simulink model "CDF_VSVPWM_Grid_Parallel"  (R2024b) (continued)
== matlab function CDF_VSVPWM_Grid_Parallel/VSVPWM/矢量作用时间计算/I3/sin(pi//6+u) ==
  data: u input; y output
function y = fcn(u)

y = sin(pi/6+u);

== matlab function CDF_VSVPWM_Grid_Parallel/VSVPWM/矢量作用时间计算/I4/sin(pi//3-u) ==
  data: u input; y output
function y = fcn(u)

y = sin(pi/3-u);

== matlab function CDF_VSVPWM_Grid_Parallel/VSVPWM/矢量作用时间计算/I4/sin(pi//6+u) ==
  data: u input; y output
function y = fcn(u)

y = sin(pi/6+u);

== matlab function CDF_VSVPWM_Grid_Parallel/VSVPWM/矢量作用时间计算/I5/sin(pi//3+u) ==
  data: u input; y output
function y = fcn(u)

y = sin(pi/3+u);

== matlab function CDF_VSVPWM_Grid_Parallel/VSVPWM/矢量作用时间计算/I5/sin(pi//6+u) ==
  data: u input; y output
function y = fcn(u)

y = sin(pi/6+u);

== matlab function CDF_VSVPWM_Grid_Parallel/VSVPWM/MATLAB Function ==
  data: n input; T1 output; N input; Ta input; Tb input; Tc input; T2 output; T3 output; T4 output; T5 output; k1 input; k2 input
function [T1,T2,T3,T4,T5] = fcn(n, N, Ta, Tb, Tc, k1, k2)
% Dual-distribution-factor timing allocation.
% k1 and k2 are clipped here to enforce the physical range in Eq. (3-35).

sector = round(N);
region = round(n);

k1 = min(1.0, max(0.0, k1));
k2 = min(1.0, max(0.0, k2));

k1_pos = k1;
k1_neg = 1.0 - k1;
k2_pos = k2;
k2_neg = 1.0 - k2;

T1 = 0.0; T2 = 0.0; T3 = 0.0; T4 = 0.0; T5 = 0.0;

switch sector
    case {1, 3, 5}
        switch region
            case 1
                T1 = k1_neg * Tb / 2.0;
                T2 = k2_neg * Ta / 2.0;
                T3 = Tc / 2.0;
                T4 = k1_pos * Tb / 2.0;
                T5 = k2_pos * Ta;
            case 2
                T1 = k1_neg * Tb / 2.0 + k1_neg * Tc / 3.0;
                T2 = k2_neg * Ta / 2.0;
                T3 = Tc / 6.0;
                T4 = k1_pos * Tb / 2.0;
                T5 = k2_pos * Ta + k2_pos * 2.0 * Tc / 3.0;
            case 3
                T1 = k1_neg * Tc / 3.0;
                T2 = k2_neg * Ta / 2.0;
                T3 = Tc / 6.0;
                T4 = Tb / 2.0;
                T5 = k2_pos * Ta + k2_pos * 2.0 * Tc / 3.0;
            case 4
                T1 = k2_neg * Ta / 2.0 + k1_neg * Tc / 3.0;
                T2 = Tb / 2.0;
                T3 = Tc / 6.0;
                T4 = k2_pos * Ta / 2.0;
                T5 = k1_pos * 2.0 * Tc / 3.0;
            case 5
                T1 = k1_neg * Tc / 3.0;
                T2 = Tb / 2.0;
                T3 = Tc / 6.0;
                T4 = Ta / 2.0;
                T5 = k1_pos * 2.0 * Tc / 3.0;
        end

    case {2, 4, 6}
        switch region
            case 1
                T1 = k1_neg * Ta / 2.0;
                T2 = k2_neg * Tb / 2.0;
                T3 = Tc / 2.0;
                T4 = k1_pos * Ta / 2.0;
                T5 = k2_pos * Tb;
            case 2
                T1 = k1_neg * Ta / 2.0 + k1_neg * Tc / 3.0;
                T2 = k2_neg * Tb / 2.0;
                T3 = Tc / 6.0;
                T4 = k1_pos * Ta / 2.0;
                T5 = k2_pos * Tb + k2_pos * 2.0 * Tc / 3.0;
            case 3
                T1 = k1_neg * Ta / 2.0 + k1_neg * Tc / 3.0;
                T2 = Tb / 2.0;
                T3 = Tc / 6.0;
                T4 = k1_pos * Ta / 2.0;
                T5 = k2_pos * 2.0 * Tc / 3.0;
            case 4
                T1 = k1_neg * Tc / 3.0;
                T2 = k1_neg * Ta / 2.0;
                T3 = Tc / 6.0;
                T4 = Tb / 2.0;
                T5 = k1_pos * Ta + k1_pos * 2.0 * Tc / 3.0;
            case 5
                T1 = k1_neg * Tc / 3.0;
                T2 = Ta / 2.0;
                T3 = Tc / 6.0;
                T4 = Tb / 2.0;
                T5 = k1_pos * 2.0 * Tc / 3.0;
        end
end
end

== matlab function CDF_VSVPWM_Grid_Parallel/VSVPWM1/矢量作用时间计算/I5/sin(pi//3+u) ==
  data: u input; y output
function y = fcn(u)

y = sin(pi/3+u);

== matlab function CDF_VSVPWM_Grid_Parallel/VSVPWM1/矢量作用时间计算/I5/sin(pi//6+u) ==
  data: u input; y output
function y = fcn(u)

y = sin(pi/6+u);

== matlab function CDF_VSVPWM_Grid_Parallel/ixiy映射 ==
  data: N input; ix output; ia input; ib input; ic input; iy output
function [ix, iy] = assign_xy_currents(N, ia, ib, ic)
% Characteristic-current mapping defined in Table 3-8.
sector = round(N);
if sector < 1 || sector > 6
    ix = 0;
    iy = 0;
    return;
end

switch sector
    case 1
        ix = -ia; iy = -ic;
    case 2
        ix =  ic; iy =  ib;
    case 3
        ix = -ib; iy = -ia;
    case 4
        ix =  ia; iy =  ic;
    case 5
        ix = -ic; iy = -ib;
    case 6
        ix =  ib; iy =  ia;
    otherwise
        ix = 0;
        iy = 0;
end
end

== matlab function CDF_VSVPWM_Grid_Parallel/VSVPWM1/生成时间调制波/Ⅰ扇区的调制信号计算/1-2/MATLAB Function2 ==
  data: unused input; tc1 output; unused1 input; T3 input; T4 input; T5 input; tc2 output
function [tc1,tc2] = fcn(~,~,T3,T4,T5)

tc2 =-(2*T3+2*T4+T5);
tc1 =0;

== matlab function CDF_VSVPWM_Grid_Parallel/VSVPWM/旧的时序逻辑分配（很好） ==
  data: n input; T1 output; N input; Ta input; Tb input; Tc input; T2 output; T3 output; T4 output; T5 output; k input
function [T1, T2,T3,T4,T5] = fcn(n,N,Ta,Tb,Tc,k)
% 这里的输入 k 必须被外部 Saturation 模块严格限幅在 [-1, 1]
% 当中点电压平衡时，k = 0

% 将零中心偏差因子 [-1, 1] 转换为正负小矢量的绝对比例系数 [0, 1]
k_pos = (1 + k) / 2; 
k_neg = (1 - k) / 2;

T1 = 0; T2 = 0; T3 = 0; T4 = 0; T5 = 0;

switch N
    case {1, 3, 5} % 奇数大扇区 (假设 T4 为正小矢量，T1/T5 为负小矢量)
        switch n
            case 1
                T1 = k_neg*Tb/2;            T2 = k_neg*Ta/2;    T3 = Tc/2;
                T4 = k_pos*Tb/2;            T5 = k_pos*Ta;
            case 2
                T1 = k_neg*Tb/2 + k_neg*Tc/3; T2 = k_neg*Ta/2;    T3 = Tc/6;
                T4 = k_pos*Tb/2;            T5 = k_pos*Ta + k_pos*2*Tc/3;
            case 3
                T1 = k_neg*Tc/3;            T2 = k_neg*Ta/2;    T3 = Tc/6;
                T4 = Tb/2;                  T5 = k_pos*Ta + k_pos*2*Tc/3;
            case 4
                T1 = k_neg*Ta/2 + k_neg*Tc/3; T2 = Tb/2;          T3 = Tc/6;
                T4 = k_pos*Ta/2;            T5 = k_pos*2*Tc/3;
            case 5
                T1 = k_neg*Tc/3;            T2 = Tb/2;          T3 = Tc/6;
                T4 = Ta/2;                  T5 = k_pos*2*Tc/3;
        end
        
    case {2, 4, 6} % 偶数大扇区
        switch n
            case 1
                T1 = k_neg*Ta/2;            T2 = k_neg*Tb/2;    T3 = Tc/2;
                T4 = k_pos*Ta/2;            T5 = k_pos*Tb;
            case 2
                T1 = k_neg*Ta/2 + k_neg*Tc/3; T2 = k_neg*Tb/2;    T3 = Tc/6;
                T4 = k_pos*Ta/2;            T5 = k_pos*Tb + k_pos*2*Tc/3;
            case 3
                T1 = k_neg*Ta/2 + k_neg*Tc/3; T2 = Tb/2;          T3 = Tc/6;
                T4 = k_pos*Ta/2;            T5 = k_pos*2*Tc/3;
            case 4
                T1 = k_neg*Tc/3;            T2 = k_neg*Ta/2;    T3 = Tc/6;
                T4 = Tb/2;                  T5 = k_pos*Ta + k_pos*2*Tc/3;
            case 5
                T1 = k_neg*Tc/3;            T2 = Ta/2;          T3 = Tc/6;
                T4 = Tb/2;                  T5 = k_pos*2*Tc/3;
        end
end

== matlab function CDF_VSVPWM_Grid_Parallel/MATLAB Function1 ==
  data: dUdc input; k1_inv1 output; iz input; ix1 input; iy1 input; k2_inv1 output
function [k1_inv1, k2_inv1] = AllocationFactorGen_Inv1(dUdc, iz, ix1, iy1)
% CDF-VSVPWM allocation factor generator.
% The iz input keeps the existing external sign preprocessing.

dU_max = 3.5;
Iz_max = 12.0;
K_np0  = 0.16;
K_z0   = 0.025;
tau    = 1.8;

Enp = abs(dUdc) / max(dU_max, eps);
Ez  = abs(iz)   / max(Iz_max, eps);
rho = 1.0 / (1.0 + exp(-tau * (Ez - Enp)));

K_z_dyn  = K_z0  * (1.0 + rho);
K_np_dyn = K_np0 * (2.0 - rho);

np_term_1 = dUdc * sign(ix1);
np_term_2 = -dUdc * sign(iy1);
z_term    = -iz;

if np_term_1 * z_term < 0
    K_np_1 = K_np_dyn;
    K_z_1  = K_z_dyn;
else
    K_np_1 = K_np0;
    K_z_1  = K_z0;
end

if np_term_2 * z_term < 0
    K_np_2 = K_np_dyn;
    K_z_2  = K_z_dyn;
else
    K_np_2 = K_np0;
    K_z_2  = K_z0;
end

k1_raw = 0.5 + K_np_1 * np_term_1 + K_z_1 * z_term;
k2_raw = 0.5 + K_np_2 * np_term_2 + K_z_2 * z_term;

k1_inv1 = min(1.0, max(0.0, k1_raw));
k2_inv1 = min(1.0, max(0.0, k2_raw));
end

== matlab function CDF_VSVPWM_Grid_Parallel/VSVPWM1/MATLAB Function ==
  data: n input; T1 output; N input; Ta input; Tb input; Tc input; T2 output; T3 output; T4 output; T5 output; k1 input; k2 input
function [T1,T2,T3,T4,T5] = fcn(n, N, Ta, Tb, Tc, k1, k2)
% Dual-distribution-factor timing allocation.
% k1 and k2 are clipped here to enforce the physical range in Eq. (3-35).

sector = round(N);
region = round(n);

k1 = min(1.0, max(0.0, k1));
k2 = min(1.0, max(0.0, k2));

k1_pos = k1;
k1_neg = 1.0 - k1;
k2_pos = k2;
k2_neg = 1.0 - k2;

T1 = 0.0; T2 = 0.0; T3 = 0.0; T4 = 0.0; T5 = 0.0;

switch sector
    case {1, 3, 5}
        switch region
            case 1
                T1 = k1_neg * Tb / 2.0;
                T2 = k2_neg * Ta / 2.0;
                T3 = Tc / 2.0;
                T4 = k1_pos * Tb / 2.0;
                T5 = k2_pos * Ta;
            case 2
                T1 = k1_neg * Tb / 2.0 + k1_neg * Tc / 3.0;
                T2 = k2_neg * Ta / 2.0;
                T3 = Tc / 6.0;
                T4 = k1_pos * Tb / 2.0;
                T5 = k2_pos * Ta + k2_pos * 2.0 * Tc / 3.0;
            case 3
                T1 = k1_neg * Tc / 3.0;
                T2 = k2_neg * Ta / 2.0;
                T3 = Tc / 6.0;
                T4 = Tb / 2.0;
                T5 = k2_pos * Ta + k2_pos * 2.0 * Tc / 3.0;
            case 4
                T1 = k2_neg * Ta / 2.0 + k1_neg * Tc / 3.0;
                T2 = Tb / 2.0;
                T3 = Tc / 6.0;
                T4 = k2_pos * Ta / 2.0;
                T5 = k1_pos * 2.0 * Tc / 3.0;
            case 5
                T1 = k1_neg * Tc / 3.0;
                T2 = Tb / 2.0;
                T3 = Tc / 6.0;
                T4 = Ta / 2.0;
                T5 = k1_pos * 2.0 * Tc / 3.0;
        end

    case {2, 4, 6}
        switch region
            case 1
                T1 = k1_neg * Ta / 2.0;
                T2 = k2_neg * Tb / 2.0;
                T3 = Tc / 2.0;
                T4 = k1_pos * Ta / 2.0;
                T5 = k2_pos * Tb;
            case 2
                T1 = k1_neg * Ta / 2.0 + k1_neg * Tc / 3.0;
                T2 = k2_neg * Tb / 2.0;
                T3 = Tc / 6.0;
                T4 = k1_pos * Ta / 2.0;
                T5 = k2_pos * Tb + k2_pos * 2.0 * Tc / 3.0;
            case 3
                T1 = k1_neg * Ta / 2.0 + k1_neg * Tc / 3.0;
                T2 = Tb / 2.0;
                T3 = Tc / 6.0;
                T4 = k1_pos * Ta / 2.0;
                T5 = k2_pos * 2.0 * Tc / 3.0;
            case 4
                T1 = k1_neg * Tc / 3.0;
                T2 = k1_neg * Ta / 2.0;
                T3 = Tc / 6.0;
                T4 = Tb / 2.0;
                T5 = k1_pos * Ta + k1_pos * 2.0 * Tc / 3.0;
            case 5
                T1 = k1_neg * Tc / 3.0;
                T2 = Ta / 2.0;
                T3 = Tc / 6.0;
                T4 = Tb / 2.0;
                T5 = k1_pos * 2.0 * Tc / 3.0;
        end
end
end

== matlab function CDF_VSVPWM_Grid_Parallel/111 ==
  data: N input; ix output; ia input; ib input; ic input; iy output
function [ix, iy] = assign_xy_currents(N, ia, ib, ic)
% Characteristic-current mapping defined in Table 3-8.
sector = round(N);
if sector < 1 || sector > 6
    ix = 0;
    iy = 0;
    return;
end

switch sector
    case 1
        ix = -ia; iy = -ic;
    case 2
        ix =  ic; iy =  ib;
    case 3
        ix = -ib; iy = -ia;
    case 4
        ix =  ia; iy =  ic;
    case 5
        ix = -ic; iy = -ib;
    case 6
        ix =  ib; iy =  ia;
    otherwise
        ix = 0;
        iy = 0;
end
end

== matlab function CDF_VSVPWM_Grid_Parallel/VSVPWM1/MATLAB Function1 ==
  data: n input; T1 output; N input; Ta input; Tb input; Tc input; T2 output; T3 output; T4 output; T5 output; k input
function [T1,T2,T3,T4,T5] = fcn(n,N,Ta,Tb,Tc,k)
% 这里的输入 k 必须被外部 Saturation 模块严格限幅在 [-1, 1]
% 当中点电压平衡时，k = 0

% 将零中心偏差因子 [-1, 1] 转换为正负小矢量的绝对比例系数 [0, 1]
k_pos = (1 + k) / 2; 
k_neg = (1 - k) / 2;

T1 = 0; T2 = 0; T3 = 0; T4 = 0; T5 = 0;

switch N
    case {1, 3, 5} % 奇数大扇区 (假设 T4 为正小矢量，T1/T5 为负小矢量)
        switch n
            case 1
                T1 = k_neg*Tb/2;            T2 = k_neg*Ta/2;    T3 = Tc/2;
                T4 = k_pos*Tb/2;            T5 = k_pos*Ta;
            case 2
                T1 = k_neg*Tb/2 + k_neg*Tc/3; T2 = k_neg*Ta/2;    T3 = Tc/6;
                T4 = k_pos*Tb/2;            T5 = k_pos*Ta + k_pos*2*Tc/3;
            case 3
                T1 = k_neg*Tc/3;            T2 = k_neg*Ta/2;    T3 = Tc/6;
                T4 = Tb/2;                  T5 = k_pos*Ta + k_pos*2*Tc/3;
            case 4
                T1 = k_neg*Ta/2 + k_neg*Tc/3; T2 = Tb/2;          T3 = Tc/6;
                T4 = k_pos*Ta/2;            T5 = k_pos*2*Tc/3;
            case 5
                T1 = k_neg*Tc/3;            T2 = Tb/2;          T3 = Tc/6;
                T4 = Ta/2;                  T5 = k_pos*2*Tc/3;
        end
        
    case {2, 4, 6} % 偶数大扇区
        switch n
            case 1
                T1 = k_neg*Ta/2;            T2 = k_neg*Tb/2;    T3 = Tc/2;
                T4 = k_pos*Ta/2;            T5 = k_pos*Tb;
            case 2
                T1 = k_neg*Ta/2 + k_neg*Tc/3; T2 = k_neg*Tb/2;    T3 = Tc/6;
                T4 = k_pos*Ta/2;            T5 = k_pos*Tb + k_pos*2*Tc/3;
            case 3
                T1 = k_neg*Ta/2 + k_neg*Tc/3; T2 = Tb/2;          T3 = Tc/6;
                T4 = k_pos*Ta/2;            T5 = k_pos*2*Tc/3;
            case 4
                T1 = k_neg*Tc/3;            T2 = k_neg*Ta/2;    T3 = Tc/6;
                T4 = Tb/2;                  T5 = k_pos*Ta + k_pos*2*Tc/3;
            case 5
                T1 = k_neg*Tc/3;            T2 = Ta/2;          T3 = Tc/6;
                T4 = Tb/2;                  T5 = k_pos*2*Tc/3;
        end
end

== matlab function CDF_VSVPWM_Grid_Parallel/VSVPWM1/生成时间调制波/Ⅰ扇区的调制信号计算/1-3/MATLAB Function ==
  data: T1 input; ta1 output; T2 input; T3 input; T4 input; unused input; ta2 output
function [ta1,ta2] = fcn(T1,T2,T3,T4,~)

ta1 =2*(T1+T2+T3+T4);
ta2 =0;

== matlab function CDF_VSVPWM_Grid_Parallel/VSVPWM1/生成时间调制波/Ⅰ扇区的调制信号计算/1-3/MATLAB Function1 ==
  data: T1 input; tb1 output; unused input; unused1 input; T4 input; T5 input; tb2 output
function [tb1,tb2] = fcn(T1,~,~,T4,T5)

tb1 =2*T1;
tb2 =-1*(2*T4+T5);

== matlab function CDF_VSVPWM_Grid_Parallel/VSVPWM1/生成时间调制波/Ⅰ扇区的调制信号计算/1-3/MATLAB Function2 ==
  data: unused input; tc1 output; unused1 input; T3 input; T4 input; T5 input; tc2 output
function [tc1,tc2] = fcn(~,~,T3,T4,T5)

tc2 =-1*(2*T3+2*T4+T5);
tc1 =0;

== matlab function CDF_VSVPWM_Grid_Parallel/VSVPWM1/生成时间调制波/Ⅰ扇区的调制信号计算/1-4/MATLAB Function ==
  data: T1 input; ta1 output; T2 input; T3 input; unused input; unused1 input; ta2 output
function [ta1,ta2] = fcn(T1,T2,T3,~,~)

ta1 =2*(T1+T2+T3);
ta2 =0;

== matlab function CDF_VSVPWM_Grid_Parallel/VSVPWM1/生成时间调制波/Ⅰ扇区的调制信号计算/1-4/MATLAB Function1 ==
  data: T1 input; tb1 output; T2 input; unused input; unused1 input; T5 input; tb2 output
function [tb1,tb2] = fcn(T1,T2,~,~,T5)

tb1 =2*(T1+T2);
tb2 =-T5;

== matlab function CDF_VSVPWM_Grid_Parallel/VSVPWM1/生成时间调制波/Ⅰ扇区的调制信号计算/1-4/MATLAB Function2 ==
  data: unused input; tc1 output; T2 input; T3 input; T4 input; T5 input; tc2 output
function [tc1,tc2] = fcn(~,T2,T3,T4,T5)

tc2 =-(2*T2+2*T3+2*T4+T5);
tc1 =0;

== matlab function CDF_VSVPWM_Grid_Parallel/VSVPWM1/生成时间调制波/Ⅰ扇区的调制信号计算/1-5/MATLAB Function ==
  data: T1 input; ta1 output; T2 input; T3 input; T4 input; unused input; ta2 output
function [ta1,ta2] = fcn(T1,T2,T3,T4,~)

ta1 =2*(T1+T2+T3+T4);
ta2 =0;

== matlab function CDF_VSVPWM_Grid_Parallel/VSVPWM1/生成时间调制波/Ⅰ扇区的调制信号计算/1-5/MATLAB Function1 ==
  data: T1 input; tb1 output; T2 input; unused input; T4 input; T5 input; tb2 output
function [tb1,tb2] = fcn(T1,T2,~,T4,T5)

tb1 =2*(T1+T2);
tb2 =-(2*T4+T5);

== matlab function CDF_VSVPWM_Grid_Parallel/VSVPWM1/生成时间调制波/Ⅰ扇区的调制信号计算/1-5/MATLAB Function2 ==
  data: unused input; tc1 output; T2 input; T3 input; T4 input; T5 input; tc2 output
function [tc1,tc2] = fcn(~,T2,T3,T4,T5)

tc2 =-(2*T2+2*T3+2*T4+T5);
tc1 =0;

== matlab function CDF_VSVPWM_Grid_Parallel/VSVPWM1/生成时间调制波/Ⅱ扇区的调制信号计算/2-1/MATLAB Function ==
  data: T1 input; ta1 output; unused input; unused1 input; unused2 input; T5 input; ta2 output
function [ta1,ta2] = fcn(T1,~,~,~,T5)

ta1 =2*T1;
ta2 =-T5;

== matlab function CDF_VSVPWM_Grid_Parallel/VSVPWM1/生成时间调制波/Ⅱ扇区的调制信号计算/2-1/MATLAB Function1 ==
  data: T1 input; tb1 output; T2 input; unused input; unused1 input; unused2 input; tb2 output
function [tb1,tb2] = fcn(T1,T2,~,~,~)

tb1 =2*(T1+T2);
tb2 =0;

== matlab function CDF_VSVPWM_Grid_Parallel/VSVPWM1/生成时间调制波/Ⅱ扇区的调制信号计算/2-1/MATLAB Function2 ==
  data: unused input; tc1 output; unused1 input; unused2 input; T4 input; T5 input; tc2 output
function [tc1,tc2] = fcn(~,~,~,T4,T5)

tc2 =-(2*T4+T5);
tc1 =0;

== matlab function CDF_VSVPWM_Grid_Parallel/VSVPWM1/生成时间调制波/Ⅱ扇区的调制信号计算/2-2/MATLAB Function ==
  data: T1 input; ta1 output; unused input; unused1 input; unused2 input; T5 input; ta2 output
function [ta1,ta2] = fcn(T1,~,~,~,T5)

ta1 =2*T1;
ta2 =-T5;

== matlab function CDF_VSVPWM_Grid_Parallel/VSVPWM1/生成时间调制波/Ⅱ扇区的调制信号计算/2-2/MATLAB Function1 ==
  data: T1 input; tb1 output; T2 input; T3 input; unused input; unused1 input; tb2 output
function [tb1,tb2] = fcn(T1,T2,T3,~,~)

tb1 =2*(T1+T2+T3);
tb2 =0;

== matlab function CDF_VSVPWM_Grid_Parallel/VSVPWM1/生成时间调制波/Ⅱ扇区的调制信号计算/2-2/MATLAB Function2 ==
  data: unused input; tc1 output; unused1 input; T3 input; T4 input; T5 input; tc2 output
function [tc1,tc2] = fcn(~,~,T3,T4,T5)

tc2 =-(2*T3+2*T4+T5);
tc1 =0;

== matlab function CDF_VSVPWM_Grid_Parallel/VSVPWM1/生成时间调制波/Ⅱ扇区的调制信号计算/2-3/MATLAB Function ==
  data: T1 input; ta1 output; T2 input; unused input; unused1 input; T5 input; ta2 output
function [ta1,ta2] = fcn(T1,T2,~,~,T5)

ta1 =2*(T1+T2);
ta2 =-T5;

== matlab function CDF_VSVPWM_Grid_Parallel/VSVPWM1/生成时间调制波/Ⅱ扇区的调制信号计算/2-3/MATLAB Function1 ==
  data: T1 input; tb1 output; T2 input; T3 input; unused input; unused1 input; tb2 output
function [tb1,tb2] = fcn(T1,T2,T3,~,~)

tb1 =2*(T1+T2+T3);
tb2 =0;

== matlab function CDF_VSVPWM_Grid_Parallel/VSVPWM1/生成时间调制波/Ⅱ扇区的调制信号计算/2-3/MATLAB Function2 ==
  data: unused input; tc1 output; T2 input; T3 input; T4 input; T5 input; tc2 output
function [tc1,tc2] = fcn(~,T2,T3,T4,T5)

tc2 =-(2*T2+2*T3+2*T4+T5);
tc1 =0;

== matlab function CDF_VSVPWM_Grid_Parallel/VSVPWM1/生成时间调制波/Ⅱ扇区的调制信号计算/2-4/MATLAB Function ==
  data: T1 input; ta1 output; unused input; unused1 input; T4 input; T5 input; ta2 output
function [ta1,ta2] = fcn(T1,~,~,T4,T5)

ta1 =2*T1;
ta2 =-(2*T4+T5);

== matlab function CDF_VSVPWM_Grid_Parallel/VSVPWM1/生成时间调制波/Ⅱ扇区的调制信号计算/2-4/MATLAB Function1 ==
  data: T1 input; tb1 output; T2 input; T3 input; T4 input; unused input; tb2 output
function [tb1,tb2] = fcn(T1,T2,T3,T4,~)

tb1 =2*(T1+T2+T3+T4);
tb2 =0;

== matlab function CDF_VSVPWM_Grid_Parallel/VSVPWM1/生成时间调制波/Ⅱ扇区的调制信号计算/2-4/MATLAB Function2 ==
  data: unused input; tc1 output; unused1 input; T3 input; T4 input; T5 input; tc2 output
function [tc1,tc2] = fcn(~,~,T3,T4,T5)

tc2 =-(2*T3+2*T4+T5);
tc1 =0;

== matlab function CDF_VSVPWM_Grid_Parallel/VSVPWM1/生成时间调制波/Ⅱ扇区的调制信号计算/2-5/MATLAB Function ==
  data: T1 input; ta1 output; T2 input; unused input; T4 input; T5 input; ta2 output
function [ta1,ta2] = fcn(T1,T2,~,T4,T5)

ta1 =2*(T1+T2);
ta2 =-(2*T4+T5);

== matlab function CDF_VSVPWM_Grid_Parallel/VSVPWM1/生成时间调制波/Ⅱ扇区的调制信号计算/2-5/MATLAB Function1 ==
  data: T1 input; tb1 output; T2 input; T3 input; T4 input; unused input; tb2 output
function [tb1,tb2] = fcn(T1,T2,T3,T4,~)

tb1 =2*(T1+T2+T3+T4);
tb2 =0;

== matlab function CDF_VSVPWM_Grid_Parallel/VSVPWM1/生成时间调制波/Ⅱ扇区的调制信号计算/2-5/MATLAB Function2 ==
  data: unused input; tc1 output; T2 input; T3 input; T4 input; T5 input; tc2 output
function [tc1,tc2] = fcn(~,T2,T3,T4,T5)

tc2 =-(2*T2+2*T3+2*T4+T5);
tc1 =0;

== matlab function CDF_VSVPWM_Grid_Parallel/VSVPWM1/生成时间调制波/Ⅲ扇区的调制信号计算1/1-1/MATLAB Function ==
  data: T1 input; ta1 output; T2 input; unused input; unused1 input; unused2 input; ta2 output
function [ta1,ta2] = fcn(T1,T2,~,~,~)

ta1 =2*(T1+T2);
ta2 =0;

== matlab function CDF_VSVPWM_Grid_Parallel/4-2 ==
  data: ealpha input; ebeta input; Ts input; ealphak1 output; ebetak1 output
function [ealphak1, ebetak1] = fcn(ealpha, ebeta, Ts)
    % 假设电网基波频率为50Hz
    w = 2*pi*50;  
    
    % 前向欧拉近似预测 k+1 时刻电网电压
    ealphak1 = ealpha - Ts*w*ebeta;
    ebetak1  = ebeta  + Ts*w*ealpha;
end

== matlab function CDF_VSVPWM_Grid_Parallel/VSVPWM1/生成时间调制波/Ⅲ扇区的调制信号计算1/1-1/MATLAB Function1 ==
  data: T1 input; tb1 output; unused input; unused1 input; unused2 input; T5 input; tb2 output
function [tb1,tb2] = fcn(T1,~,~,~,T5)

tb1 =2*T1;
tb2 =-T5;

== matlab function CDF_VSVPWM_Grid_Parallel/VSVPWM1/生成时间调制波/Ⅲ扇区的调制信号计算1/1-1/MATLAB Function2 ==
  data: unused input; tc1 output; unused1 input; unused2 input; T4 input; T5 input; tc2 output
function [tc1,tc2] = fcn(~,~,~,T4,T5)

tc2 =-(2*T4+T5);
tc1 =0;

== matlab function CDF_VSVPWM_Grid_Parallel/VSVPWM1/生成时间调制波/Ⅲ扇区的调制信号计算1/1-2/MATLAB Function ==
  data: T1 input; ta1 output; T2 input; T3 input; unused input; unused1 input; ta2 output
function [ta1,ta2] = fcn(T1,T2,T3,~,~)

ta1 =2*(T1+T2+T3);
ta2 =0;

== matlab function CDF_VSVPWM_Grid_Parallel/VSVPWM1/生成时间调制波/Ⅲ扇区的调制信号计算1/1-2/MATLAB Function1 ==
  data: T1 input; tb1 output; unused input; unused1 input; unused2 input; T5 input; tb2 output
function [tb1,tb2] = fcn(T1,~,~,~,T5)

tb1 =2*T1;
tb2 =-T5;

== matlab function CDF_VSVPWM_Grid_Parallel/VSVPWM1/生成时间调制波/Ⅲ扇区的调制信号计算1/1-2/MATLAB Function2 ==
  data: unused input; tc1 output; unused1 input; T3 input; T4 input; T5 input; tc2 output
function [tc1,tc2] = fcn(~,~,T3,T4,T5)

tc2 =-(2*T3+2*T4+T5);
tc1 =0;

== matlab function CDF_VSVPWM_Grid_Parallel/VSVPWM1/生成时间调制波/Ⅲ扇区的调制信号计算1/1-3/MATLAB Function ==
  data: T1 input; ta1 output; T2 input; T3 input; T4 input; unused input; ta2 output
function [ta1,ta2] = fcn(T1,T2,T3,T4,~)

ta1 =2*(T1+T2+T3+T4);
ta2 =0;

== matlab function CDF_VSVPWM_Grid_Parallel/VSVPWM1/生成时间调制波/Ⅲ扇区的调制信号计算1/1-3/MATLAB Function1 ==
  data: T1 input; tb1 output; unused input; unused1 input; T4 input; T5 input; tb2 output
function [tb1,tb2] = fcn(T1,~,~,T4,T5)

tb1 =2*T1;
tb2 =-1*(2*T4+T5);

== matlab function CDF_VSVPWM_Grid_Parallel/VSVPWM1/生成时间调制波/Ⅲ扇区的调制信号计算1/1-3/MATLAB Function2 ==
  data: unused input; tc1 output; unused1 input; T3 input; T4 input; T5 input; tc2 output
function [tc1,tc2] = fcn(~,~,T3,T4,T5)

tc2 =-1*(2*T3+2*T4+T5);
tc1 =0;

== matlab function CDF_VSVPWM_Grid_Parallel/4-1 ==
  data: ealpha input; ebeta input; Ts input; ealphak1 output; ebetak1 output
function [ealphak1, ebetak1] = fcn(ealpha, ebeta, Ts)
    % 假设电网基波频率为50Hz
    w = 2*pi*50;  
    
    % 前向欧拉近似预测 k+1 时刻电网电压
    ealphak1 = ealpha - Ts*w*ebeta;
    ebetak1  = ebeta  + Ts*w*ealpha;
end

== matlab function CDF_VSVPWM_Grid_Parallel/VSVPWM1/生成时间调制波/Ⅲ扇区的调制信号计算1/1-4/MATLAB Function ==
  data: T1 input; ta1 output; T2 input; T3 input; unused input; unused1 input; ta2 output
function [ta1,ta2] = fcn(T1,T2,T3,~,~)

ta1 =2*(T1+T2+T3);
ta2 =0;

== matlab function CDF_VSVPWM_Grid_Parallel/VSVPWM1/生成时间调制波/Ⅲ扇区的调制信号计算1/1-4/MATLAB Function1 ==
  data: T1 input; tb1 output; T2 input; unused input; unused1 input; T5 input; tb2 output
function [tb1,tb2] = fcn(T1,T2,~,~,T5)

tb1 =2*(T1+T2);
tb2 =-T5;

== matlab function CDF_VSVPWM_Grid_Parallel/VSVPWM1/生成时间调制波/Ⅲ扇区的调制信号计算1/1-4/MATLAB Function2 ==
  data: unused input; tc1 output; T2 input; T3 input; T4 input; T5 input; tc2 output
function [tc1,tc2] = fcn(~,T2,T3,T4,T5)

tc2 =-(2*T2+2*T3+2*T4+T5);
tc1 =0;

== matlab function CDF_VSVPWM_Grid_Parallel/VSVPWM1/生成时间调制波/Ⅲ扇区的调制信号计算1/1-5/MATLAB Function ==
  data: T1 input; ta1 output; T2 input; T3 input; T4 input; unused input; ta2 output
function [ta1,ta2] = fcn(T1,T2,T3,T4,~)

ta1 =2*(T1+T2+T3+T4);
ta2 =0;

== matlab function CDF_VSVPWM_Grid_Parallel/VSVPWM1/生成时间调制波/Ⅲ扇区的调制信号计算1/1-5/MATLAB Function1 ==
  data: T1 input; tb1 output; T2 input; unused input; T4 input; T5 input; tb2 output
function [tb1,tb2] = fcn(T1,T2,~,T4,T5)

tb1 =2*(T1+T2);
tb2 =-(2*T4+T5);

== matlab function CDF_VSVPWM_Grid_Parallel/VSVPWM1/生成时间调制波/Ⅲ扇区的调制信号计算1/1-5/MATLAB Function2 ==
  data: unused input; tc1 output; T2 input; T3 input; T4 input; T5 input; tc2 output
function [tc1,tc2] = fcn(~,T2,T3,T4,T5)

tc2 =-(2*T2+2*T3+2*T4+T5);
tc1 =0;

== matlab function CDF_VSVPWM_Grid_Parallel/VSVPWM/大扇区判断/MATLAB Function ==
  data: u input; y output
function y = fcn(u)

y = u*3/pi;

== matlab function CDF_VSVPWM_Grid_Parallel/VSVPWM/大扇区判断/MATLAB Function1 ==
  data: u input; y output
function y = fcn(u)

y = u;

== matlab function CDF_VSVPWM_Grid_Parallel/VSVPWM/大扇区判断/MATLAB Function2 ==
  data: u input; y output
function y = fcn(u)

y = u-pi/3;

== matlab function CDF_VSVPWM_Grid_Parallel/VSVPWM/大扇区判断/MATLAB Function3 ==
  data: u input; y output
function y = fcn(u)

y = u-2*pi/3;
